simulink model "wlan_phy_rx_pmd"  (Simulink 7.8)
settings: Solver=VariableStepDiscrete  SolverMode=SingleTasking  StartTime=0.0  StopTime=rx_sim.sim_time  RelTol=1e-3
PostLoadFcn:
  | wlan_phy_rx_init
InitFcn:
  | wlan_phy_rx_init
StartFcn:
  | wlan_phy_rx_init
values from neighbouring files (symbols this model uses):
  barker_seq20 = 0.95 * circshift(barker_seq20, [0 4]) ./ max(abs(barker_seq20))    [wlan_phy_rx_init.m]
  bit_scrambler_lfsr_bytes = sum(bit_scrambler_lfsr_bytes .* repmat(2.^[0:7], 127, 1),2)    [wlan_phy_rx_init.m]
  longCorr_coef_i = [3*real(longCorr_coef)]    [wlan_phy_rx_init.m]
  longCorr_coef_q = [3*imag(longCorr_coef)]    [wlan_phy_rx_init.m]
  MAX_CP_LEN = 32    [wlan_phy_rx_init.m]
  MAX_NCBPS = NCBPS_11n_64QAM    (= 312)    [wlan_phy_rx_init.m]
  MAX_NUM_BYTES = 2^16-1    (= 65535)    [wlan_phy_rx_init.m]
  MAX_NUM_SC = 64    [wlan_phy_rx_init.m]
  MAX_NUM_SYMS = 600    [wlan_phy_rx_init.m]
  NDBPS_11a_BPSK12 = 24    [wlan_phy_rx_init.m]
  preFFT_sampBuff_numSamps = 4*MAX_NUM_SC    (= 256)    [wlan_phy_rx_init.m]
  rx_sim = struct()    [wlan_phy_rx_init.m]
  scr_ind_rev = zeros(1,128)    [wlan_phy_rx_init.m]
undefined symbols (not defined in the file or in its folder): gcbh, mcs_rom_11ag, mcs_rom_11n, wlan_rx_deinterleave_rom_gen, xlBlockLoadCallback, xlGetNormalizedPeriod, xlipgetpartsetting
libraries: xbsIndex_r4 x4943, xbsEDKLib_r4 x1
(part 5 of 28)

== system wlan_phy_rx_pmd/CFO & Samp Buffer/Samp Buffer ==
  note: Circular sample buffer records all incoming I/Q samples post pkt det
      | Samples are unloaded from the buffer after LTS sync and fed into
      | the FFT (via CFO correction and a FIFO)
      |
      | This buffer is required because 802.11 uses the same preamble samples
      | (the LTS) for sample-level synchronization and CFO estimation. The
      | received LTS samples must be saved and CFO-corrected before the
      | FFT to avoid degrading the channel estimates.
  Pkt Det : Inport
  Pkt Done : Inport  Port=2
  rx I : Inport  Port=3
  rx Q : Inport  Port=4
  IQ valid : Inport  Port=5
  LTS Sync 2 : Inport  Port=6
  FIFO tready : Inport  Port=7
  Circular Sample Buffer : xbsIndex_r4/Dual Port RAM  depth=preFFT_sampBuff_numSamps  initVector=1 * repmat([0:63], 1, 4)  distributed_mem=Block RAM  init_a=0  init_b=0  rst_a=off  rst_b=off  en_a=off  en_b=off  latency=1  write_mode_A=Read After Write  write_mode_B=Read After Write  dbl_ovrd=off  optimize=Area  block_type=dpram
  Concat : Subsystem
  Constant3 : xbsIndex_r4/Constant  const=0  gui_display_data_type=Fixed-point  arith_type=Unsigned  n_bits=32  bin_pt=0  preci_type=Single  exp_width=8  frac_width=24  explicit_period=off  period=8  equ=P=C  opselect=C  inp2=PCIN>>17  opr=+  inp1=P  carry=CIN  dbl_ovrd=off  block_type=constant
  Constant4 : xbsIndex_r4/Constant  const=0  gui_display_data_type=Boolean  arith_type=Boolean  n_bits=6  bin_pt=0  preci_type=Single  exp_width=8  frac_width=24  explicit_period=off  period=8  equ=P=C  opselect=C  inp2=PCIN>>17  opr=+  inp1=P  carry=CIN  dbl_ovrd=off  block_type=constant
  Counter : xbsIndex_r4/Counter  cnt_type=Free Running  cnt_to=Inf  operation=Up  start_count=0  cnt_by_val=1  arith_type=Unsigned  n_bits=ceil(log2(preFFT_sampBuff_numSamps))  bin_pt=0  load_pin=off  rst=off  en=on  explicit_period=off  period=8  dbl_ovrd=off  use_behavioral_HDL=off  implementation=Fabric  block_type=counter
  Delay : xbsIndex_r4/Delay  rst=off  infoeditControl=Selection of Reset will increase slice count due to use of real FFs and instead of SRLs  en=off  latency=1  dbl_ovrd=off  reg_retiming=off  block_type=delay
  Delay2 : xbsIndex_r4/Delay  rst=off  infoeditControl=Selection of Reset will increase slice count due to use of real FFs and instead of SRLs  en=off  latency=1  dbl_ovrd=off  reg_retiming=off  block_type=delay
  FFT Load Ctrl : Subsystem
  FSM : Subsystem
  Gateway Out{1..9} (9 alike) : xbsIndex_r4/Gateway Out  inherit_from_input=off  hdl_port=off  timing_constraint=None  locs_specified=off  LOCs={}  UseAsDAC=off  DACChannel='1'  block_type=gatewayout
  Logical2 : xbsIndex_r4/Logical  logical_function=AND  inputs=2  en=off  latency=0  precision=Full  arith_type=Unsigned  n_bits=16  bin_pt=0  align_bp=on  dbl_ovrd=off  block_type=logical
  Posedge : Subsystem
  Posedge3 : Subsystem
  Register : xbsIndex_r4/Register  init=0  rst=off  en=off  dbl_ovrd=off  block_type=register
  Register1 : xbsIndex_r4/Register  init=0  rst=off  en=off  dbl_ovrd=off  block_type=register
  S-R Latch : Subsystem
  Scope : Scope  NumInputPorts=9
      settings: TimeRange=10000  YMin=-0.4~-0.4~0~0~0~-0.1~-0.1~-5~-5  YMax=0.5~0.4~275~275~4000~1.1~1.1~5~5  SaveName=ScopeData25
  Slice : Subsystem
  Terminator : Terminator
  dbg_payload : xbsIndex_r4/Gateway Out  inherit_from_input=off  hdl_port=on  timing_constraint=None  locs_specified=off  LOCs={}  UseAsDAC=off  DACChannel='1'  block_type=gatewayout
  I : Outport
  Q : Outport  Port=2
  Buff Rd : Outport  Port=3
  " IQ valid" : Outport  Port=4
  tlast : Outport  Port=5
  tag scope: OFDM_RX_RUNNING_DSSS_BLOCK=global
  wires:
  Concat/1(IQ) -> Circular Sample Buffer/2
  Circular Sample Buffer/1 -> Terminator/1
  FFT Load Ctrl/1(Rd Addr) -> Circular Sample Buffer/4, Gateway Out1/1
  Constant3/1 -> Circular Sample Buffer/5
  Constant4/1 -> Circular Sample Buffer/6
  Logical2/1 -> Posedge/1(D)
  Posedge/1(Q) -> FFT Load Ctrl/3(LTS Sync), FSM/2(LTS)
  FFT Load Ctrl/5(FFT Load Done) -> FSM/1(FFT Load Done), Gateway Out7/1
  FSM/3(FFT Data load) -> FFT Load Ctrl/6(FFT Load)
  Circular Sample Buffer/2 -> Slice/1(IQ)
  FSM/1(FFT skip sel) -> FFT Load Ctrl/4(Skip Sel)
  FFT Load Ctrl/3(FFT tvalid) -> Delay/1, Posedge3/1(D)
  FFT Load Ctrl/2(Reading) -> Delay2/1
  Pkt Done/1 -> FSM/3(Pkt Done), S-R Latch/2(R), Gateway Out4/1
  rx I/1 -> Concat/1(I), Gateway Out6/1
  rx Q/1 -> Concat/2(Q)
  IQ valid/1 -> Circular Sample Buffer/3, Counter/1
  Slice/1(I) -> I/1, Gateway Out5/1
  Slice/2(Q) -> Q/1
  FIFO tready/1 -> FFT Load Ctrl/2(data tready)
  Delay2/1 -> Buff Rd/1
  Delay/1 -> " IQ valid"/1, Gateway Out8/1
  FFT Load Ctrl/4(FFT tlast) -> tlast/1
  LTS Sync 2/1 -> Logical2/1
  Pkt Det/1 -> Logical2/2, Gateway Out3/1
  Gateway Out5/1 -> Scope/2  "FFT In I"
  Gateway Out1/1 -> Scope/3  "Rd Addr"
  Gateway Out2/1 -> Scope/4  "Wr Addr"
  Gateway Out3/1 -> Scope/5  "Pkt Det"
  Posedge3/1(Q) -> S-R Latch/1(S)
  S-R Latch/1(Q) -> Register/1, [OFDM_RX_RUNNING_DSSS_BLOCK], Gateway Out9/1
  Gateway Out4/1 -> Scope/6  "Pkt Done"
  Gateway Out6/1 -> Scope/1  "ADC I"
  Register/1 -> Register1/1
  Register1/1 -> dbg_payload/1
  Gateway Out7/1 -> Scope/7  "FFT Load Done"
  Counter/1 -> Circular Sample Buffer/1, FFT Load Ctrl/1(Wr Addr), Gateway Out2/1
  FSM/2(FFT LTS load) -> FFT Load Ctrl/5(FFT LTS Load)
  Gateway Out8/1 -> Scope/8  "FFT In tvalid"
  Gateway Out9/1 -> Scope/9  "DEBUG Payload"

== system wlan_phy_rx_pmd/Chan Est & EQ/Chan Est ==
  x_re : Inport
  x_im : Inport  Port=2
  x_ind : Inport  Port=3
  x_valid : Inport  Port=4
  Sym Ind : Inport  Port=5
  Abs : Abs
  Abs1 : Abs
  Chan Est Offload : Subsystem
  Constant : xbsIndex_r4/Constant  const=0.4625  gui_display_data_type=Fixed-point  arith_type=Signed (2's comp)  n_bits=19  bin_pt=17  preci_type=Single  exp_width=8  frac_width=24  explicit_period=on  period=1  equ=P=C  opselect=C  inp2=PCIN>>17  opr=+  inp1=P  carry=CIN  dbl_ovrd=off  block_type=constant
  Constant1 : xbsIndex_r4/Constant  const=0  gui_display_data_type=Fixed-point  arith_type=Signed (2's comp)  n_bits=19  bin_pt=17  preci_type=Single  exp_width=8  frac_width=24  explicit_period=on  period=1  equ=P=C  opselect=C  inp2=PCIN>>17  opr=+  inp1=P  carry=CIN  dbl_ovrd=off  block_type=constant
  Control : Subsystem
  Delay{1..5} (5 alike) : xbsIndex_r4/Delay  rst=off  infoeditControl=Selection of Reset will increase slice count due to use of real FFs and instead of SRLs  en=off  latency=6  dbl_ovrd=off  reg_retiming=off  block_type=delay
      settings: xl_area=[0, 0, 0, 0, 0, 0, 0]  block_version=VER_STRING_GOES_HERE
  Estimate Buffers : Subsystem
  Gateway Out{1..8,12..13} (10 alike) : xbsIndex_r4/Gateway Out  inherit_from_input=off  hdl_port=off  timing_constraint=None  locs_specified=off  LOCs={}  UseAsDAC=off  DACChannel='1'  block_type=gatewayout
  H Est Outputs : Scope  NumInputPorts=9
      settings: TimeRange=50000  YMin=-1~-1~-0.5~-0.5~0~0~0~0~0  YMax=1~1~1~0.5~80~60~1~1~0.8  SaveName=ScopeData16
  Inverter : xbsIndex_r4/Inverter  en=off  latency=0  dbl_ovrd=off  block_type=inv
  Logical1 : xbsIndex_r4/Logical  logical_function=AND  inputs=2  en=off  latency=0  precision=Full  arith_type=Unsigned  n_bits=16  bin_pt=0  align_bp=on  dbl_ovrd=off  block_type=logical
  Logical2 : xbsIndex_r4/Logical  logical_function=AND  inputs=2  en=off  latency=0  precision=Full  arith_type=Unsigned  n_bits=16  bin_pt=0  align_bp=on  dbl_ovrd=off  block_type=logical
  Mult by LTF : Subsystem
  Real-Imag to Complex : RealImagToComplex
  Real-Imag to Complex1 : RealImagToComplex
  Real-Imag to Complex2 : RealImagToComplex
  To Float : Subsystem
  To Workspace : ToWorkspace  VariableName=pre_eq  SampleTime=-1  SaveFormat=Array
  To Workspace1 : ToWorkspace  VariableName=pre_eq_valid  SampleTime=-1  SaveFormat=Array
  " x_I" : Outport
  " x_Q" : Outport  Port=2
  H_I : Outport  Port=3
  H_Q : Outport  Port=4
  " valid" : Outport  Port=5
  " x_ind" : Outport  Port=6
  " sym_ind" : Outport  Port=7
  wires:
  Logical2/1 -> Estimate Buffers/4(Est_WE)
  x_valid/1 -> Mult by LTF/3(x_valid), Logical1/1, Logical2/1
  Sym Ind/1 -> Control/1(Sym Ind), Delay2/1
  Control/2(Est Overwrite) -> Estimate Buffers/5(First Sym)
  Control/1(Est WrEn) -> Logical2/2
  Logical1/1 -> Delay1/1
  Inverter/1 -> Logical1/2
  Estimate Buffers/2(h_Q[n]) -> Gateway Out3/1, To Float/4("H_Q "), Chan Est Offload/3(x_Q)
  Gateway Out8/1 -> Real-Imag to Complex/1, H Est Outputs/1  "x_I"
  Gateway Out1/1 -> Real-Imag to Complex/2, H Est Outputs/2  "x_Q"
  Gateway Out2/1 -> Real-Imag to Complex1/1, H Est Outputs/3  "H_I"
  Gateway Out3/1 -> Real-Imag to Complex1/2, H Est Outputs/4  "H_Q"
  Gateway Out4/1 -> H Est Outputs/5  "SC Ind"
  Gateway Out5/1 -> H Est Outputs/7  "Valid Out"
  x_ind/1 -> Estimate Buffers/7(Est_Addr), Delay5/1, Mult by LTF/4(x_ind)
  x_re/1 -> Mult by LTF/1(x_re), Delay3/1
  x_im/1 -> Mult by LTF/2(x_im), Delay4/1
  Mult by LTF/2(H_im) -> Estimate Buffers/2(x_Q), Gateway Out1/1, Gateway Out13/1
  Mult by LTF/1(H_re) -> Estimate Buffers/1(x_I), Gateway Out8/1, Gateway Out12/1
  To Float/1(" x_I") -> " x_I"/1
  To Float/2(" x_Q") -> " x_Q"/1
  To Float/3(H_I) -> H_I/1
  To Float/4(" H_Q") -> H_Q/1
  To Float/5(" x_ind") -> " x_ind"/1
  To Float/6(" x_valid") -> " valid"/1
  To Float/7(" sym_ind") -> " sym_ind"/1
  Abs/1 -> H Est Outputs/9
  Real-Imag to Complex/1 -> Abs/1
  Real-Imag to Complex1/1 -> Abs1/1
  Abs1/1 -> H Est Outputs/8
  Gateway Out6/1 -> H Est Outputs/6  "Sym Ind"
  Control/3(Scale) -> Estimate Buffers/6(Scale)
  Control/4(Sym Invalid) -> Inverter/1
  Estimate Buffers/1(h_I[n]) -> Gateway Out2/1, To Float/3("H_I "), Chan Est Offload/2(x_I)
  Mult by LTF/3(Empty Sc) -> Estimate Buffers/3(Empty Sc)
  Delay5/1 -> Gateway Out4/1, To Float/5(x_ind), Chan Est Offload/1(x_ind)
  Delay1/1 -> Chan Est Offload/4(x_valid), To Float/6(x_valid), Gateway Out5/1, Gateway Out7/1
  Delay2/1 -> To Float/7(sym_ind), Chan Est Offload/5(sym_ind), Gateway Out6/1
  Delay4/1 -> To Float/2("x_Q ")
  Delay3/1 -> To Float/1("x_I ")
  Gateway Out12/1 -> Real-Imag to Complex2/1
  Gateway Out13/1 -> Real-Imag to Complex2/2
  Real-Imag to Complex2/1 -> To Workspace/1
  Gateway Out7/1 -> To Workspace1/1

== system wlan_phy_rx_pmd/Chan Est & EQ/Debug Outputs ==
  note: Only capture data and pilot subcarriers
      | for debug outputs and chipscope
  I : Inport
  Q : Inport  Port=2
  valid : Inport  Port=3
  x_ind : Inport  Port=4
  4x Buffer : Subsystem
  Constant : xbsIndex_r4/Constant  const=0  gui_display_data_type=Fixed-point  arith_type=Unsigned  n_bits=1  bin_pt=0  preci_type=Single  exp_width=8  frac_width=24  explicit_period=off  period=1  equ=P=C  opselect=C  inp2=PCIN>>17  opr=+  inp1=P  carry=CIN  dbl_ovrd=off  block_type=constant
  Constant1 : xbsIndex_r4/Constant  const=27  gui_display_data_type=Fixed-point  arith_type=Unsigned  n_bits=5  bin_pt=0  preci_type=Single  exp_width=8  frac_width=24  explicit_period=off  period=1  equ=P=C  opselect=C  inp2=PCIN>>17  opr=+  inp1=P  carry=CIN  dbl_ovrd=off  block_type=constant
  Constant2 : xbsIndex_r4/Constant  const=37  gui_display_data_type=Fixed-point  arith_type=Unsigned  n_bits=6  bin_pt=0  preci_type=Single  exp_width=8  frac_width=24  explicit_period=off  period=1  equ=P=C  opselect=C  inp2=PCIN>>17  opr=+  inp1=P  carry=CIN  dbl_ovrd=off  block_type=constant
  Constant3 : xbsIndex_r4/Constant  const=29  gui_display_data_type=Fixed-point  arith_type=Unsigned  n_bits=5  bin_pt=0  preci_type=Single  exp_width=8  frac_width=24  explicit_period=off  period=1  equ=P=C  opselect=C  inp2=PCIN>>17  opr=+  inp1=P  carry=CIN  dbl_ovrd=off  block_type=constant
  Constant4 : xbsIndex_r4/Constant  const=35  gui_display_data_type=Fixed-point  arith_type=Unsigned  n_bits=6  bin_pt=0  preci_type=Single  exp_width=8  frac_width=24  explicit_period=off  period=1  equ=P=C  opselect=C  inp2=PCIN>>17  opr=+  inp1=P  carry=CIN  dbl_ovrd=off  block_type=constant
  Debug EQ IQ : Scope  NumInputPorts=7
      settings: YMin=-1.1~-1~-1.1~-1~-5~-5~-5  YMax=1.1~1~1.2~1~5~5~5  SaveName=ScopeData13
  Delay : xbsIndex_r4/Delay  rst=off  infoeditControl=Selection of Reset will increase slice count due to use of real FFs and instead of SRLs  en=off  latency=1  dbl_ovrd=off  reg_retiming=off  block_type=delay
  Delay1 : xbsIndex_r4/Delay  rst=off  infoeditControl=Selection of Reset will increase slice count due to use of real FFs and instead of SRLs  en=off  latency=1  dbl_ovrd=off  reg_retiming=off  block_type=delay
  Gateway Out12 : xbsIndex_r4/Gateway Out  inherit_from_input=off  hdl_port=off  timing_constraint=None  locs_specified=off  LOCs={}  UseAsDAC=off  DACChannel='1'  block_type=gatewayout
  Gateway Out13 : xbsIndex_r4/Gateway Out  inherit_from_input=off  hdl_port=off  timing_constraint=None  locs_specified=off  LOCs={}  UseAsDAC=off  DACChannel='1'  block_type=gatewayout
  Gateway Out8 : xbsIndex_r4/Gateway Out  inherit_from_input=off  hdl_port=off  timing_constraint=None  locs_specified=off  LOCs={}  UseAsDAC=off  DACChannel='1'  block_type=gatewayout
  Inverter1 : xbsIndex_r4/Inverter  en=off  latency=0  dbl_ovrd=off  block_type=inv
  Logical : xbsIndex_r4/Logical  logical_function=AND  inputs=3  en=off  latency=0  precision=Full  arith_type=Unsigned  n_bits=16  bin_pt=0  align_bp=on  dbl_ovrd=off  block_type=logical
  Logical1 : xbsIndex_r4/Logical  logical_function=OR  inputs=2  en=off  latency=0  precision=Full  arith_type=Unsigned  n_bits=16  bin_pt=0  align_bp=on  dbl_ovrd=off  block_type=logical
  Logical2 : xbsIndex_r4/Logical  logical_function=OR  inputs=2  en=off  latency=0  precision=Full  arith_type=Unsigned  n_bits=16  bin_pt=0  align_bp=on  dbl_ovrd=off  block_type=logical
  Mux : xbsIndex_r4/Mux  inputs=2  en=off  latency=0  precision=Full  arith_type=Unsigned  n_bits=16  bin_pt=14  quantization=Truncate  overflow=Wrap  dbl_ovrd=off  block_type=mux
  Real-Imag to Complex : RealImagToComplex
  Register{,1..2,7..8} (5 alike) : xbsIndex_r4/Register  init=0  rst=off  en=off  dbl_ovrd=off  block_type=register
  Register{3..6} (4 alike) : xbsIndex_r4/Register  init=0  rst=on  en=off  dbl_ovrd=off  block_type=register
  Relational : xbsIndex_r4/Relational  mode=a!=b  en=off  latency=0  dbl_ovrd=off  block_type=relational
  Relational1 : xbsIndex_r4/Relational  mode=a<b  en=off  latency=0  dbl_ovrd=off  block_type=relational
  Relational2 : xbsIndex_r4/Relational  mode=a>b  en=off  latency=0  dbl_ovrd=off  block_type=relational
  Relational3 : xbsIndex_r4/Relational  mode=a<b  en=off  latency=0  dbl_ovrd=off  block_type=relational
  Relational4 : xbsIndex_r4/Relational  mode=a>b  en=off  latency=0  dbl_ovrd=off  block_type=relational
  To FP1 : xbsIndex_r4/Convert  gui_display_data_type=Fixed-point  arith_type="Signed  (2's comp)"  n_bits=14  bin_pt=13  float_type=Single  exp_bits=8  fraction_bits=24  quantization=Truncate  overflow=Saturate  en=off  latency=0  dbl_ovrd=off  pipeline=off  block_type=convert
  To FP4 : xbsIndex_r4/Convert  gui_display_data_type=Fixed-point  arith_type="Signed  (2's comp)"  n_bits=14  bin_pt=13  float_type=Single  exp_bits=8  fraction_bits=24  quantization=Truncate  overflow=Saturate  en=off  latency=0  dbl_ovrd=off  pipeline=off  block_type=convert
  To Workspace : ToWorkspace  VariableName=eq_out  SampleTime=-1  SaveFormat=Array
  To Workspace1 : ToWorkspace  VariableName=eq_out_valid  SampleTime=-1  SaveFormat=Array
  dbg_EQ_I : xbsIndex_r4/Gateway Out  inherit_from_input=off  hdl_port=on  timing_constraint=None  locs_specified=off  LOCs={}  UseAsDAC=off  DACChannel='1'  block_type=gatewayout
  dbg_EQ_Q : xbsIndex_r4/Gateway Out  inherit_from_input=off  hdl_port=on  timing_constraint=None  locs_specified=off  LOCs={}  UseAsDAC=off  DACChannel='1'  block_type=gatewayout
  dbg_EQ_Q{1..4} (4 alike) : xbsIndex_r4/Gateway Out  inherit_from_input=off  hdl_port=off  timing_constraint=None  locs_specified=off  LOCs={}  UseAsDAC=off  DACChannel='1'  block_type=gatewayout
  tag scope: CS_EQ_I=global, CS_EQ_Q=global, CS_EQ_Valid=global
  wires:
  Inverter1/1 -> Register3/2, Register6/2
  Logical/1 -> Inverter1/1, Delay/1, Gateway Out8/1, 4x Buffer/3(Vin)
  Register2/1 -> To FP1/1, Register6/1, Gateway Out13/1
  Register1/1 -> To FP4/1, Register3/1, Gateway Out12/1
  x_ind/1 -> Register/1
  Constant/1 -> Relational/2
  Relational/1 -> Logical/2
  I/1 -> Register1/1
  Q/1 -> Register2/1
  Constant1/1 -> Relational1/2
  Constant2/1 -> Relational2/2
  Relational1/1 -> Logical1/1
  Relational2/1 -> Logical1/2
  Register3/1 -> [CS_EQ_I], dbg_EQ_Q3/1
  Register6/1 -> [CS_EQ_Q], dbg_EQ_Q1/1
  Delay/1 -> [CS_EQ_Valid], dbg_EQ_Q2/1
  valid/1 -> Delay1/1
  Delay1/1 -> Logical/1
  Register7/1 -> dbg_EQ_I/1
  Register8/1 -> dbg_EQ_Q/1
  dbg_EQ_I/1 -> Debug EQ IQ/3
  dbg_EQ_Q/1 -> Debug EQ IQ/4
  dbg_EQ_Q3/1 -> Debug EQ IQ/5
  dbg_EQ_Q1/1 -> Debug EQ IQ/6
  dbg_EQ_Q2/1 -> Debug EQ IQ/7
  Gateway Out12/1 -> Real-Imag to Complex/1
  Gateway Out13/1 -> Real-Imag to Complex/2
  Real-Imag to Complex/1 -> To Workspace/1
  Gateway Out8/1 -> To Workspace1/1
  To FP4/1 -> 4x Buffer/1(I)
  To FP1/1 -> 4x Buffer/2(Q)
  Register4/1 -> Register7/1
  4x Buffer/1(" I") -> Register4/1
  4x Buffer/3(#Vout) -> Register4/2, Register5/2, dbg_EQ_Q4/1
  4x Buffer/2(" Q") -> Register5/1
  Register5/1 -> Register8/1
  dbg_EQ_Q4/1 -> Debug EQ IQ/2
  Constant3/1 -> Relational3/2
  Constant4/1 -> Relational4/2
  Relational3/1 -> Logical2/1
  Relational4/1 -> Logical2/2
  Register/1 -> Relational/1, Relational1/1, Relational2/1, Relational3/1, Relational4/1
  Mux/1 -> Logical/3
  [RX_PHY_MODE_11N_AC] -> Mux/1
  Logical2/1 -> Mux/3
  Logical1/1 -> Mux/2

== system wlan_phy_rx_pmd/Chan Est & EQ/Equalizer, Pilot Processing & PHY Mode Det ==
  note: PHY Mode affects many subsystems - use Goto/From
      | tags to avoid messy routing of these control signals
  x_I : Inport
  x_Q : Inport  Port=2
  H_I : Inport  Port=3
  H_Q : Inport  Port=4
  x_valid : Inport  Port=5
  x_ind : Inport  Port=6
  Sym ind : Inport  Port=7
  Constant : xbsIndex_r4/Constant  const=0.034  gui_display_data_type=Fixed-point  arith_type=Signed (2's comp)  n_bits=16  bin_pt=13  preci_type=Single  exp_width=8  frac_width=24  explicit_period=on  period=1  equ=P=C  opselect=C  inp2=PCIN>>17  opr=+  inp1=P  carry=CIN  dbl_ovrd=off  block_type=constant
  Constant1 : xbsIndex_r4/Constant  const=0  gui_display_data_type=Fixed-point  arith_type=Signed (2's comp)  n_bits=16  bin_pt=13  preci_type=Single  exp_width=8  frac_width=24  explicit_period=on  period=1  equ=P=C  opselect=C  inp2=PCIN>>17  opr=+  inp1=P  carry=CIN  dbl_ovrd=off  block_type=constant
  Convert : xbsIndex_r4/Convert  gui_display_data_type=Fixed-point  arith_type="Signed  (2's comp)"  n_bits=16  bin_pt=14  float_type=Single  exp_bits=8  fraction_bits=24  quantization="Round  (unbiased: +/- Inf)"  overflow=Saturate  en=off  latency=1  dbl_ovrd=off  pipeline=off  block_type=convert
  Convert1 : xbsIndex_r4/Convert  gui_display_data_type=Fixed-point  arith_type="Signed  (2's comp)"  n_bits=16  bin_pt=14  float_type=Single  exp_bits=8  fraction_bits=24  quantization="Round  (unbiased: +/- Inf)"  overflow=Saturate  en=off  latency=1  dbl_ovrd=off  pipeline=off  block_type=convert
  Convert2 : xbsIndex_r4/Convert  gui_display_data_type=Fixed-point  arith_type="Signed  (2's comp)"  n_bits=20  bin_pt=14  float_type=Single  exp_bits=8  fraction_bits=24  quantization="Round  (unbiased: +/- Inf)"  overflow=Saturate  en=off  latency=1  dbl_ovrd=off  pipeline=off  block_type=convert
  Convert4 : xbsIndex_r4/Convert  gui_display_data_type=Fixed-point  arith_type="Signed  (2's comp)"  n_bits=20  bin_pt=14  float_type=Single  exp_bits=8  fraction_bits=24  quantization="Round  (unbiased: +/- Inf)"  overflow=Saturate  en=off  latency=1  dbl_ovrd=off  pipeline=off  block_type=convert
  Delay{1,3..4,8,10} (5 alike) : xbsIndex_r4/Delay  rst=off  infoeditControl=Selection of Reset will increase slice count due to use of real FFs and instead of SRLs  en=off  latency=62  dbl_ovrd=off  reg_retiming=off  block_type=delay
  Delay12 : xbsIndex_r4/Delay  rst=off  infoeditControl=Selection of Reset will increase slice count due to use of real FFs and instead of SRLs  en=off  latency=23  dbl_ovrd=off  reg_retiming=off  block_type=delay
  Delay14 : xbsIndex_r4/Delay  rst=off  infoeditControl=Selection of Reset will increase slice count due to use of real FFs and instead of SRLs  en=off  latency=23  dbl_ovrd=off  reg_retiming=off  block_type=delay
  Delay17 : xbsIndex_r4/Delay  rst=off  infoeditControl=Selection of Reset will increase slice count due to use of real FFs and instead of SRLs  en=off  latency=23  dbl_ovrd=off  reg_retiming=off  block_type=delay
  Delay2 : xbsIndex_r4/Delay  rst=off  infoeditControl=Selection of Reset will increase slice count due to use of real FFs and instead of SRLs  en=off  latency=8  dbl_ovrd=off  reg_retiming=off  block_type=delay
  Delay6 : xbsIndex_r4/Delay  rst=off  infoeditControl=Selection of Reset will increase slice count due to use of real FFs and instead of SRLs  en=off  latency=8  dbl_ovrd=off  reg_retiming=off  block_type=delay
  Delay7 : xbsIndex_r4/Delay  rst=off  infoeditControl=Selection of Reset will increase slice count due to use of real FFs and instead of SRLs  en=off  latency=8  dbl_ovrd=off  reg_retiming=off  block_type=delay
  Divide : xbsIndex_r4/Divide  flow_control=NonBlocking  axi_optimize_goal=Resources  algorithm_type=High_Radix  infoedit1=Specify output fraction-width for Fixed-point division.  fractional_width=16  latency=21  rate=1  has_a_tlast=off  has_a_tuser=off  has_b_tlast=off  has_b_tuser=off  en=off  has_result_tready=off  result_tlast_behv=Pass_A_TLAST  has_underflow=off  has_overflow=off  has_invalid_op=off  has_divide_by_zero=off  fpo_op_type=mergeddivide  block_type=divide
      settings: infoedit=Blocking: Wait for data on all inputs, allow back-pressure.<br>NonBlocking: operate every cycle in which all inputs are valid, no back-pressure.
  Divide1 : xbsIndex_r4/Divide  flow_control=NonBlocking  axi_optimize_goal=Resources  algorithm_type=High_Radix  infoedit1=Specify output fraction-width for Fixed-point division.  fractional_width=16  latency=21  rate=1  has_a_tlast=off  has_a_tuser=off  has_b_tlast=off  has_b_tuser=off  en=off  has_result_tready=off  result_tlast_behv=Pass_A_TLAST  has_underflow=off  has_overflow=off  has_invalid_op=off  has_divide_by_zero=off  fpo_op_type=mergeddivide  block_type=divide
      settings: infoedit=Blocking: Wait for data on all inputs, allow back-pressure.<br>NonBlocking: operate every cycle in which all inputs are valid, no back-pressure.
  EQ : Scope  NumInputPorts=8
      settings: TimeRange=20000  YMin=-0.5~-0.5~0~0~0~-2~-2~0  YMax=0.5~0.5~1~80~40~2~0.5~1  SaveName=ScopeData9
  Gateway Out{,1..7} (8 alike) : xbsIndex_r4/Gateway Out  inherit_from_input=off  hdl_port=off  timing_constraint=None  locs_specified=off  LOCs={}  UseAsDAC=off  DACChannel='1'  block_type=gatewayout
  Logical4 : xbsIndex_r4/Logical  logical_function=OR  inputs=2  en=off  latency=0  precision=Full  arith_type=Unsigned  n_bits=16  bin_pt=0  align_bp=on  dbl_ovrd=off  block_type=logical
  Mag : Subsystem
  PHY Mode Det : Subsystem
  Phase Err Calc : Subsystem
  Register3 : xbsIndex_r4/Register  init=0  rst=off  en=off  dbl_ovrd=off  block_type=register
  Register4 : xbsIndex_r4/Register  init=0  rst=off  en=off  dbl_ovrd=off  block_type=register
  Register5 : xbsIndex_r4/Register  init=0  rst=off  en=off  dbl_ovrd=off  block_type=register
  a*conj(b) : Subsystem
  " x_I" : Outport
  " x_Q" : Outport  Port=2
  " x_valid" : Outport  Port=3
  phase err : Outport  Port=4
  phase err vld : Outport  Port=5
  " x_ind" : Outport  Port=6
  " sym ind" : Outport  Port=7
  tag scope: CS_Phase_Err=global, RX_PHY_MODE_11AC=global, RX_PHY_MODE_11AG=global, RX_PHY_MODE_11N=global, RX_PHY_MODE_11N_AC=global
  wires:
  x_ind/1 -> Delay2/1
  Sym ind/1 -> Gateway Out6/1, Delay7/1
  x_I/1 -> a*conj(b)/1(A_I), Gateway Out/1
  x_Q/1 -> a*conj(b)/2(A_Q), Gateway Out1/1
  H_I/1 -> a*conj(b)/3(B_I), Mag/1(I)
  H_Q/1 -> a*conj(b)/4(B_Q), Mag/2(Q)
  x_valid/1 -> Gateway Out4/1, Delay6/1
  Phase Err Calc/1(Sym Phase Err) -> phase err/1, [CS_Phase_Err]
  Delay7/1 -> Phase Err Calc/5(sym_ind), Delay17/1
  a*conj(b)/1(P_I) -> Phase Err Calc/1(CP_I), Register4/1
  a*conj(b)/2(P_Q) -> Phase Err Calc/2(CP_Q), Register3/1
  Delay2/1 -> Phase Err Calc/4(x_ind), Delay14/1
  Mag/1(M) -> Register5/1
  Divide/1 -> Convert/1, Convert2/1
  Divide1/1 -> Convert1/1, Convert4/1
  Convert/1 -> Delay8/1, PHY Mode Det/1(x_I)
  Convert1/1 -> Delay10/1, PHY Mode Det/2(x_Q)
  Register3/1 -> Divide1/1
  Register4/1 -> Divide/1
  Delay12/1 -> Delay4/1, PHY Mode Det/3(x_valid)
  Delay14/1 -> Delay1/1
  Delay17/1 -> Delay3/1, PHY Mode Det/4(Sym ind)
  Delay6/1 -> Phase Err Calc/3(x_valid), Delay12/1
  Gateway Out6/1 -> EQ/5  "Sym Ind"
  Gateway Out5/1 -> EQ/4  "EQ X Ind"
  Gateway Out4/1 -> EQ/3  "X Valid"
  Gateway Out2/1 -> EQ/7  "EQ Q"
  Gateway Out1/1 -> EQ/2  "X_Q"
  Gateway Out/1 -> EQ/1  "X_I"
  Gateway Out7/1 -> EQ/6  "EQ I"
  Gateway Out3/1 -> EQ/8  "EQ Valid"
  Register5/1 -> Divide/2, Divide1/2
  PHY Mode Det/3(11a/g) -> [RX_PHY_MODE_11AG]
  PHY Mode Det/2(11n) -> [RX_PHY_MODE_11N], Logical4/2
  PHY Mode Det/1(11ac) -> [RX_PHY_MODE_11AC], Logical4/1
  Logical4/1 -> [RX_PHY_MODE_11N_AC]
  Delay8/1 -> " x_I"/1, Gateway Out7/1
  Delay10/1 -> " x_Q"/1, Gateway Out2/1
  Delay4/1 -> " x_valid"/1, Gateway Out3/1
  Delay1/1 -> " x_ind"/1, Gateway Out5/1
  Delay3/1 -> " sym ind"/1
  Phase Err Calc/2(phase err valid) -> phase err vld/1
  Convert2/1 -> ?
  Convert4/1 -> ?

== system wlan_phy_rx_pmd/Chan Est & EQ/Phase Tracking & Correction ==
  x_I : Inport
  x_Q : Inport  Port=2
  x_valid : Inport  Port=3
  Phase Err : Inport  Port=4
  Phase Err Valid : Inport  Port=5
  "x_ind " : Inport  Port=6
  "sym_ind " : Inport  Port=7
  Bool Safe : Subsystem
  Bool Safe1 : Subsystem
  Phase Rotation : Subsystem
  " x_I" : Outport
  " x_Q" : Outport  Port=2
  " x_valid" : Outport  Port=3
  x_ind : Outport  Port=4
  " sym ind" : Outport  Port=5
  wires:
  x_I/1 -> Phase Rotation/1(x_I)
  x_Q/1 -> Phase Rotation/2(x_Q)
  x_valid/1 -> Phase Rotation/3(x_valid)
  Phase Err/1 -> Phase Rotation/4(Phase)
  Phase Err Valid/1 -> Phase Rotation/5(Phase Valid)
  "x_ind "/1 -> Phase Rotation/6("x_ind ")
  "sym_ind "/1 -> Phase Rotation/7("sym_ind ")
  Phase Rotation/1(" x_I") -> Bool Safe/1(A)
  Phase Rotation/2(" x_Q") -> Bool Safe1/1(A)
  Phase Rotation/3(" x_valid") -> " x_valid"/1
  Phase Rotation/4(x_ind) -> x_ind/1
  Phase Rotation/5(" sym ind") -> " sym ind"/1
  Bool Safe/1(B) -> " x_I"/1
  Bool Safe1/1(B) -> " x_Q"/1

== system wlan_phy_rx_pmd/DSSS Rx/Demod ==
  Start : Inport
  I : Inport  Port=2
  Q : Inport  Port=3
  Bit-to-Byte : Subsystem
  DBPSK Demod : Subsystem
  DSSS Rx : Scope  NumInputPorts=7
      settings: TimeRange=56900  YMin=-1~-1~0.95~0~47.5~0~0  YMax=1~1~1.05~1~80~1~250
  De-spread : Subsystem
  Descramble : Subsystem
  Gateway Out{1..7} (7 alike) : xbsIndex_r4/Gateway Out  inherit_from_input=off  hdl_port=off  timing_constraint=None  locs_specified=off  LOCs={}  UseAsDAC=off  DACChannel='1'  block_type=gatewayout
  Header CRC : Subsystem
  Register8 : xbsIndex_r4/Register  init=0  rst=off  en=on  dbl_ovrd=off  block_type=register
  Sync : Subsystem
  Byte : Outport
  Byte Valid : Outport  Port=2
  Byte Ind : Outport  Port=3
  CRC Calc : Outport  Port=4
  tag scope: CS_DSSS_BIT_VALID=global
  wires:
  Q/1 -> De-spread/2(Q), Gateway Out3/1
  Start/1 -> De-spread/3(Sym Start), Gateway Out6/1
  I/1 -> De-spread/1(I), Gateway Out2/1
  De-spread/1(IQ Valid) -> DBPSK Demod/1(IQ Valid)
  De-spread/2(" I") -> DBPSK Demod/2(I)
  De-spread/3(" Q") -> DBPSK Demod/3(Q)
  DBPSK Demod/1(bit) -> Descramble/1(Bit)
  DBPSK Demod/2(bit valid) -> Descramble/2(Bit Valid)
  Descramble/1(" Bit") -> Register8/1, Sync/1(Bit)
  Descramble/2(" Bit Valid") -> Sync/2(Bit Valid), Register8/2, [CS_DSSS_BIT_VALID]
  Gateway Out2/1 -> DSSS Rx/1
  Gateway Out3/1 -> DSSS Rx/2
  Gateway Out6/1 -> DSSS Rx/3
  Gateway Out7/1 -> DSSS Rx/4
  Gateway Out1/1 -> DSSS Rx/5
  Gateway Out4/1 -> DSSS Rx/6
  Gateway Out5/1 -> DSSS Rx/7
  Sync/1(Payload Bit) -> Bit-to-Byte/1(Bit), Header CRC/1(Bit)
  Sync/2(" Bit Valid") -> Bit-to-Byte/2(Bit Valid), Header CRC/2(Bit Valid)
  Bit-to-Byte/3(Byte Ind) -> Byte Ind/1, Gateway Out1/1
  Bit-to-Byte/1(Byte) -> Byte/1, Gateway Out5/1
  Bit-to-Byte/2(Byte Valid) -> Byte Valid/1, Gateway Out4/1
  Register8/1 -> Gateway Out7/1
  Header CRC/1(CRC Calc) -> CRC Calc/1

== system wlan_phy_rx_pmd/DSSS Rx/Reset Gen ==
  note: Extend the DSSS reset event long enough to flush
      | the DSSS PHY pipeline and to reduce the
      | chance of spurious double-detections
  Reset : Inport
  Constant1 : xbsIndex_r4/Constant  const=127  gui_display_data_type=Fixed-point  arith_type=Unsigned  n_bits=7  bin_pt=0  preci_type=Single  exp_width=8  frac_width=24  explicit_period=off  period=1  equ=P=C  opselect=C  inp2=PCIN>>17  opr=+  inp1=P  carry=CIN  dbl_ovrd=off  block_type=constant
      settings: block_version=VER_STRING_GOES_HERE
  Counter1 : xbsIndex_r4/Counter  cnt_type=Free Running  cnt_to=Inf  operation=Up  start_count=0  cnt_by_val=1  arith_type=Unsigned  n_bits=7  bin_pt=0  load_pin=off  rst=on  en=on  explicit_period=off  period=1  dbl_ovrd=off  use_behavioral_HDL=off  implementation=Fabric  block_type=counter
  Down Sample3 : xbsIndex_r4/Down Sample  sample_ratio=8  sample_phase="Last Value of Frame  (most efficient)"  en=off  latency=1  dbl_ovrd=off  block_type=dsamp
  Inverter2 : xbsIndex_r4/Inverter  en=off  latency=0  dbl_ovrd=off  block_type=inv
  Logical1 : xbsIndex_r4/Logical  logical_function=OR  inputs=2  en=off  latency=0  precision=Full  arith_type=Unsigned  n_bits=8  bin_pt=2  align_bp=on  dbl_ovrd=off  block_type=logical
      settings: xl_area=[1 0 0 1 0 0 0]  block_version=VER_STRING_GOES_HERE
  Logical2 : xbsIndex_r4/Logical  logical_function=OR  inputs=2  en=off  latency=0  precision=Full  arith_type=Unsigned  n_bits=8  bin_pt=2  align_bp=on  dbl_ovrd=off  block_type=logical
      settings: xl_area=[1 0 0 1 0 0 0]  block_version=VER_STRING_GOES_HERE
  Posedge : Subsystem
  Relational1 : xbsIndex_r4/Relational  mode=a=b  en=off  latency=0  dbl_ovrd=off  block_type=relational
  S-R Latch : Subsystem
  S-R Latch2 : Subsystem
  negedge : Subsystem
  DSSS Rx Reset : Outport
  wires:
  Posedge/1(Q) -> S-R Latch/1(S), Logical1/1
  S-R Latch/1(Q) -> Logical2/2
  Counter1/1 -> Relational1/1
  Constant1/1 -> Relational1/2
  negedge/1(Q) -> S-R Latch2/1(S)
  S-R Latch2/1(Q) -> Counter1/2, Inverter2/1
  Relational1/1 -> S-R Latch2/2(R), S-R Latch/2(R)
  Inverter2/1 -> Logical1/2
  Reset/1 -> Posedge/1(D), negedge/1(D), Logical2/1
  Down Sample3/1 -> DSSS Rx Reset/1
  Logical1/1 -> Counter1/1
  Logical2/1 -> Down Sample3/1

== system wlan_phy_rx_pmd/DSSS Rx/S-R Latch1 ==
  S : Inport
  R : Inport  Port=2
  Constant1 : xbsIndex_r4/Constant  const=1  gui_display_data_type=Boolean  arith_type=Boolean  n_bits=1  bin_pt=0  preci_type=Single  exp_width=8  frac_width=24  explicit_period=off  period=1  equ=P=C  opselect=C  inp2=PCIN>>17  opr=+  inp1=P  carry=CIN  dbl_ovrd=off  block_type=constant
      settings: block_version=VER_STRING_GOES_HERE
  Convert1 : xbsIndex_r4/Convert  gui_display_data_type=Boolean  arith_type=Boolean  n_bits=1  bin_pt=0  float_type=Single  exp_bits=8  fraction_bits=24  quantization=Truncate  overflow=Wrap  en=off  latency=0  dbl_ovrd=off  pipeline=off  block_type=convert
  Convert2 : xbsIndex_r4/Convert  gui_display_data_type=Boolean  arith_type=Boolean  n_bits=1  bin_pt=0  float_type=Single  exp_bits=8  fraction_bits=24  quantization=Truncate  overflow=Wrap  en=off  latency=0  dbl_ovrd=off  pipeline=off  block_type=convert
  Register2 : xbsIndex_r4/Register  init=0  rst=on  en=on  dbl_ovrd=off  block_type=register
      settings: xl_area=[1 1 0 0 0 0 0]  block_version=VER_STRING_GOES_HERE
  Q : Outport
  wires:
  S/1 -> Convert2/1
  Register2/1 -> Q/1
  R/1 -> Convert1/1
  Constant1/1 -> Register2/1
  Convert1/1 -> Register2/2
  Convert2/1 -> Register2/3

== system wlan_phy_rx_pmd/DSSS Rx/SIGNAL Dec ==
  note: LENGTH*8 + 6 - 1:
      | Length in usec = Number of bits
      | *8: Convert to bytes
      | +6: Add SIGNAL, SERVICE and CRC bytes
      | -1: Downstream logic requires zero-indexed length
      | to compare vs. byte index
  Byte : Inport
  Byte Valid : Inport  Port=2
  Byte Ind : Inport  Port=3
  CRC Calc : Inport  Port=4
  AddSub : xbsIndex_r4/AddSub  mode=Addition  use_carryin=off  use_carryout=off  en=off  latency=0  precision=User Defined  arith_type=Unsigned  n_bits=ceil(log2(MAX_NUM_BYTES))  bin_pt=0  quantization=Truncate  overflow=Wrap  dbl_ovrd=off  use_behavioral_HDL=off  hw_selection=Fabric  pipelined=off  block_type=addsub
  Concat : xbsIndex_r4/Concat  num_inputs=2  dbl_ovrd=off  block_type=concat
  Concat1 : xbsIndex_r4/Concat  num_inputs=2  dbl_ovrd=off  block_type=concat
  Constant1 : xbsIndex_r4/Constant  const=2  gui_display_data_type=Fixed-point  arith_type=Unsigned  n_bits=2  bin_pt=0  preci_type=Single  exp_width=8  frac_width=24  explicit_period=off  period=1  equ=P=C  opselect=C  inp2=PCIN>>17  opr=+  inp1=P  carry=CIN  dbl_ovrd=off  block_type=constant
  Constant2 : xbsIndex_r4/Constant  const=0  gui_display_data_type=Fixed-point  arith_type=Unsigned  n_bits=2  bin_pt=0  preci_type=Single  exp_width=8  frac_width=24  explicit_period=off  period=1  equ=P=C  opselect=C  inp2=PCIN>>17  opr=+  inp1=P  carry=CIN  dbl_ovrd=off  block_type=constant
  Constant3 : xbsIndex_r4/Constant  const=10  gui_display_data_type=Fixed-point  arith_type=Unsigned  n_bits=8  bin_pt=0  preci_type=Single  exp_width=8  frac_width=24  explicit_period=off  period=1  equ=P=C  opselect=C  inp2=PCIN>>17  opr=+  inp1=P  carry=CIN  dbl_ovrd=off  block_type=constant
  Constant4 : xbsIndex_r4/Constant  const=3  gui_display_data_type=Fixed-point  arith_type=Unsigned  n_bits=2  bin_pt=0  preci_type=Single  exp_width=8  frac_width=24  explicit_period=off  period=1  equ=P=C  opselect=C  inp2=PCIN>>17  opr=+  inp1=P  carry=CIN  dbl_ovrd=off  block_type=constant
  Constant6 : xbsIndex_r4/Constant  const=4  gui_display_data_type=Fixed-point  arith_type=Unsigned  n_bits=3  bin_pt=0  preci_type=Single  exp_width=8  frac_width=24  explicit_period=off  period=1  equ=P=C  opselect=C  inp2=PCIN>>17  opr=+  inp1=P  carry=CIN  dbl_ovrd=off  block_type=constant
  Constant7 : xbsIndex_r4/Constant  const=5  gui_display_data_type=Fixed-point  arith_type=Unsigned  n_bits=3  bin_pt=0  preci_type=Single  exp_width=8  frac_width=24  explicit_period=off  period=1  equ=P=C  opselect=C  inp2=PCIN>>17  opr=+  inp1=P  carry=CIN  dbl_ovrd=off  block_type=constant
  Constant9 : xbsIndex_r4/Constant  const=5  gui_display_data_type=Fixed-point  arith_type=Unsigned  n_bits=3  bin_pt=0  preci_type=Single  exp_width=8  frac_width=24  explicit_period=off  period=8  equ=P=C  opselect=C  inp2=PCIN>>17  opr=+  inp1=P  carry=CIN  dbl_ovrd=off  block_type=constant
  DOUT{,2,4..5} (4 alike) : xbsIndex_r4/Register  init=0  rst=on  en=on  dbl_ovrd=off  block_type=register
  DOUT3 : xbsIndex_r4/Register  init=4  rst=on  en=on  dbl_ovrd=off  block_type=register
  DSSS SIGNAL Decode : Scope  NumInputPorts=7
      settings: TimeRange=14500  YMin=0~0~972.8~0.95~-1~-1~-1  YMax=10~1~1075.2~1.05~1~1~1  SaveName=ScopeData44
  Delay1 : xbsIndex_r4/Delay  rst=off  infoeditControl=Selection of Reset will increase slice count due to use of real FFs and instead of SRLs  en=off  latency=1  dbl_ovrd=off  reg_retiming=off  block_type=delay
  Display : Display
  Display1 : Display
  Gateway Out{,1..7} (8 alike) : xbsIndex_r4/Gateway Out  inherit_from_input=off  hdl_port=off  timing_constraint=None  locs_specified=off  LOCs={}  UseAsDAC=off  DACChannel='1'  block_type=gatewayout
  Inverter1 : xbsIndex_r4/Inverter  en=off  latency=0  dbl_ovrd=off  block_type=inv
  Length Check : Subsystem
  Logical{,1..3,7..10} (8 alike) : xbsIndex_r4/Logical  logical_function=AND  inputs=2  en=off  latency=0  precision=Full  arith_type=Unsigned  n_bits=16  bin_pt=0  align_bp=on  dbl_ovrd=off  block_type=logical
  Logical6 : xbsIndex_r4/Logical  logical_function=OR  inputs=3  en=off  latency=0  precision=Full  arith_type=Unsigned  n_bits=16  bin_pt=0  align_bp=on  dbl_ovrd=off  block_type=logical
  Relational{1..2,4,6..7} (5 alike) : xbsIndex_r4/Relational  mode=a=b  en=off  latency=0  dbl_ovrd=off  block_type=relational
  Relational3 : xbsIndex_r4/Relational  mode=a!=b  en=off  latency=0  dbl_ovrd=off  block_type=relational
  Relational8 : xbsIndex_r4/Relational  mode=a!=b  en=off  latency=0  dbl_ovrd=off  block_type=relational
  Shift : xbsIndex_r4/Shift  shift_dir=Right  shift_bits=3  en=off  latency=0  precision=Full  arith_type="Signed  (2's comp)"  n_bits=16  bin_pt=14  quantization=Truncate  overflow=Wrap  dbl_ovrd=off  block_type=shift
  LENGTH : Outport
  Valid Header : Outport  Port=2
  Error : Outport  Port=3
  wires:
  Constant2/1 -> Relational2/2
  Relational1/1 -> Logical/2
  Relational2/1 -> Logical2/2
  Logical/1 -> DOUT2/3
  Logical2/1 -> DOUT/3
  Byte/1 -> DOUT/1, DOUT2/1, DOUT3/1, DOUT4/1, DOUT5/1, Gateway Out2/1
  [DSSS_RX_RESET] -> DOUT/2, DOUT2/2, DOUT3/2, DOUT4/2, DOUT5/2
  Byte Valid/1 -> Logical2/1, Logical/1, Logical3/1, Logical8/1, Logical7/1, Gateway Out3/1
  Constant1/1 -> Relational1/2
  Byte Ind/1 -> Relational1/1, Relational4/1, Relational7/1, Relational6/1, Relational2/1
  DOUT/1 -> Relational3/1, Gateway Out1/1
  Constant3/1 -> Relational3/2
  Relational4/1 -> Logical3/2
  Constant4/1 -> Relational4/2
  Logical3/1 -> DOUT3/3
  DOUT2/1 -> Concat/2
  DOUT3/1 -> Concat/1
  Concat/1 -> Gateway Out/1, Shift/1, Gateway Out6/1, Length Check/1(Len)
  Length Check/1(Invalid) -> Logical6/2
  Relational3/1 -> Logical6/1
  Gateway Out/1 -> Display/1
  Gateway Out1/1 -> Display1/1
  Constant6/1 -> Relational6/2
  Logical8/1 -> DOUT4/3, Delay1/1
  Relational6/1 -> Logical7/2
  Relational7/1 -> Logical8/2
  Constant7/1 -> Relational7/2
  DOUT4/1 -> Concat1/1
  DOUT5/1 -> Concat1/2
  Concat1/1 -> Relational8/1
  CRC Calc/1 -> Relational8/2
  Relational8/1 -> Logical9/1, Gateway Out7/1
  Delay1/1 -> Logical9/2, Logical1/2, Logical10/2
  Logical9/1 -> Logical6/3
  Logical7/1 -> DOUT5/3
  Constant9/1 -> AddSub/2
  AddSub/1 -> LENGTH/1
  Logical6/1 -> Inverter1/1, Logical1/1
  Inverter1/1 -> Logical10/1
  Logical10/1 -> Valid Header/1, Gateway Out4/1
  Logical1/1 -> Error/1, Gateway Out5/1
  Shift/1 -> AddSub/1
  Gateway Out2/1 -> DSSS SIGNAL Decode/1
  Gateway Out3/1 -> DSSS SIGNAL Decode/2
  Gateway Out4/1 -> DSSS SIGNAL Decode/6
  Gateway Out5/1 -> DSSS SIGNAL Decode/7
  Gateway Out6/1 -> DSSS SIGNAL Decode/3
  Gateway Out7/1 -> DSSS SIGNAL Decode/4

== system wlan_phy_rx_pmd/DSSS Rx/Sym Sync ==
  En : Inport
  Rx I : Inport  Port=2
  Rx Q : Inport  Port=3
  ASR : xbsIndex_r4/Addressable Shift Register  infer_latency=on  depth=2  initVector=[0]  en=off  dbl_ovrd=off  use_behavioral_HDL=off  Optimization=Resource  block_type=addrsr
      settings: infoedit="Delay of configurable length.  Any element in the delay line can be addressed and driven onto the output port.<br><br>Hardware notes: This block is implemented using SRL16s. If Virtex-4 or Spartan-3 device is used, multiple SRLC16s are cascaded together."
  Abs : Subsystem
  DSSS Corr : Scope  NumInputPorts=7
      settings: TimeRange=14500  YMin=-1~-7.5~0~0~0~0~-1  YMax=1~7.5~35~1~1~1~1
  Display : Display
  Display1 : Display
  Down Sample1 : xbsIndex_r4/Down Sample  sample_ratio=8  sample_phase="Last Value of Frame  (most efficient)"  en=off  latency=1  dbl_ovrd=off  block_type=dsamp
  Down Sample2 : xbsIndex_r4/Down Sample  sample_ratio=8  sample_phase="Last Value of Frame  (most efficient)"  en=off  latency=1  dbl_ovrd=off  block_type=dsamp
  "FIR Compiler 6.3 " : xbsIndex_r4/FIR Compiler 6.3   coefficientvector=fliplr(barker_seq20)  coefficient_sets=1  coefficient_reload=off  filter_type=Single_Rate  rate_change_type=Integer  interpolation_rate=1  decimation_rate=1  zero_pack_factor=1  channel_sequence=Basic  number_channels=1  pattern_list='P4-0,P4-1,P4-2,P4-3,P4-4'  number_paths=2  ratespecification=Maximum_Possible  sampleperiod=8  hardwareoversamplingrate=1  coefficient_sign=Signed  quantization=Maximize_Dynamic_Range  coefficient_width=16  bestprecision=on  coefficient_fractional_bits=15  coefficient_structure=Inferred  output_rounding_mode=Truncate_LSBs  output_width=16  filter_architecture=Systolic_Multiply_Accumulate  optimization_goal=Area  data_buffer_type=Automatic  coefficient_buffer_type=Automatic  input_buffer_type=Automatic  output_buffer_type=Automatic  preference_for_other_storage=Automatic  multi_column_support=Automatic  columnconfig='20'  inter_column_pipe_length=4  data_has_tlast=Not_Required  m_data_has_tready=off  s_data_has_fifo=off  s_data_has_tuser=Not_Required  m_data_has_tuser=Not_Required  s_config_sync_mode=On_Vector  s_config_method=Single  num_reload_slots=1  has_aclken=off  has_aresetn=off  infoeditControl=ARESETn must be asserted for a minimum of 2 cycles  trim_axipin_name=on  passband_min=0.0  passband_max=0.5  stopband_min=0.5  stopband_max=1.0  filter_selection=1  gui_behaviour=Sysgen_GUI  data_sign=Signed  data_width=16  data_fractional_bits=0  data_tuser_width=1  wrapper_available=true  ip_wrap_arbitrary_binary_point=true  ip_name=FIR Compiler  ip_version=6.3  dsptool_ready=true  ipcore_usecache=true  ipcore_useipmodelcache=true  ipcore_xco_need_fpga_part=true  port_translation_map={ 'aclken' => 'en', 'aresetn' => 'rst' }  run_core_at_system_period=true  ipcore_fpga_part=xlipgetpartsetting(gcb, {'virtex6', 'xc6vlx75t', '-3', 'ff784'})  block_type=fir_compiler_v6_3
      settings: infoedit=Maximum Possible : Maximizes oversampling rate. Abstracted input handshake (no s_data_tvalid) and automatic rate propagation.<br>Hardware Oversampling Rate : Specifies oversampling rate relative to the input sample period. Abstracted input handshake (no s_data_tvalid) and automatic rate propagation.<br>Sample Period : Specifies absolute oversampling rate. No input handshake abstraction (exposes s_data_tvalid) and no rate propagation.
  Gateway Out{,1..7} (8 alike) : xbsIndex_r4/Gateway Out  inherit_from_input=off  hdl_port=off  timing_constraint=None  locs_specified=off  LOCs={}  UseAsDAC=off  DACChannel='1'  block_type=gatewayout
  Posedge : Subsystem
  Relational : xbsIndex_r4/Relational  mode=a<b  en=off  latency=0  dbl_ovrd=off  block_type=relational
  S-R Latch : Subsystem
  Sym Match : Subsystem
  Sym Sync Timeout : Subsystem
  Sym : Outport
  Timeout : Outport  Port=2
  wires:
  Rx Q/1 -> Gateway Out/1, "FIR Compiler 6.3 "/2
  Gateway Out/1 -> DSSS Corr/1
  "FIR Compiler 6.3 "/2 -> Abs/1(Valid)
  "FIR Compiler 6.3 "/3 -> Abs/2(I)
  "FIR Compiler 6.3 "/4 -> Abs/3(Q), Gateway Out1/1
  Gateway Out1/1 -> DSSS Corr/2
  Abs/1(A) -> Relational/2, Gateway Out2/1
  [regRx_DSSS_RX_CODE_CORR_THRESH] -> Down Sample2/1
  Rx I/1 -> "FIR Compiler 6.3 "/1
  Relational/1 -> ASR/1
  Gateway Out2/1 -> DSSS Corr/3
  Gateway Out3/1 -> Display/1
  [regRx_DSSS_RX_DESPREAD_DLY] -> Down Sample1/1
  Gateway Out5/1 -> DSSS Corr/4
  ? -> DSSS Corr/5
  Gateway Out6/1 -> DSSS Corr/6
  Gateway Out7/1 -> DSSS Corr/7
  Down Sample1/1 -> ASR/2, Gateway Out4/1
  Down Sample2/1 -> Relational/1, Gateway Out3/1
  ASR/1 -> Sym Match/1(Corr)
  En/1 -> Sym Match/2(En), Gateway Out6/1, Sym Sync Timeout/2(Det)
  Sym Match/1(Sym) -> Gateway Out7/1, Posedge/1(D)
  Gateway Out4/1 -> Display1/1
  Posedge/1(Q) -> S-R Latch/1(S)
  [DSSS_RX_RESET] -> S-R Latch/2(R)
  S-R Latch/1(Q) -> Sym/1, Sym Sync Timeout/1(Sym)
  Sym Sync Timeout/1(Timeout) -> Timeout/1, Gateway Out5/1

== system wlan_phy_rx_pmd/Detect & Decode/De-Interleave ==
  LLR Valid : Inport
  LLR Vec : Inport  Port=2
  Demod cfg : Inport  Port=3
  mod_sel : Inport  Port=4
  Reset : Inport  Port=5
  Mem Sel : Subsystem
  RAMs : Subsystem
  Read Addr Gen : Subsystem
  Register : xbsIndex_r4/Register  init=0  rst=on  en=on  dbl_ovrd=off  block_type=register
  Write Addr Gen : Subsystem
  b0 : xbsIndex_r4/Slice  nbits=1  boolean_output=on  mode=Lower Bit Location + Width  bit1=0  base1=MSB of Input  bit0=0  base0=LSB of Input  dbl_ovrd=off  block_type=slice
  " LLR A" : Outport
  " LLR B" : Outport  Port=2
  " tvalid" : Outport  Port=3
  wires:
  Demod cfg/1 -> b0/1
  LLR Valid/1 -> Write Addr Gen/1(tvalid)
  Reset/1 -> Write Addr Gen/3(Reset), Read Addr Gen/5(Reset), Mem Sel/1(Reset), Register/2
  b0/1 -> Write Addr Gen/2(SIGNAL Sym), Register/1
  Write Addr Gen/3(Block Wr Done) -> Mem Sel/2(En), Read Addr Gen/1(Block Wr Done), Register/3
  mod_sel/1 -> Read Addr Gen/2(mod_sel)
  [OFDM_RX_DATA_Code_Rate] -> Read Addr Gen/4(rate_sel)
  Mem Sel/1(Rd) -> RAMs/1(Rd Sel)
  Mem Sel/2(Wr) -> RAMs/2(Wr Sel)
  Write Addr Gen/1(Wr Addr A) -> RAMs/3(Wr Addr A)
  Write Addr Gen/2(Wr En) -> RAMs/4(Wr En)
  Read Addr Gen/1(Rd Addr A) -> RAMs/5(Rd Addr A)
  Read Addr Gen/2(Rd Addr B) -> RAMs/6(Rd Addr B)
  Read Addr Gen/3(Skip A) -> RAMs/7(Skip A)
  Read Addr Gen/4(Skip B) -> RAMs/8(Skip B)
  Read Addr Gen/5(Vout) -> RAMs/9(Vout)
  LLR Vec/1 -> RAMs/10(LLR Vec)
  RAMs/1(" LLR A") -> " LLR A"/1
  RAMs/2(" LLR B") -> " LLR B"/1
  RAMs/3(" tvalid") -> " tvalid"/1
  Register/1 -> Read Addr Gen/3(SIGNAL sym)
